simulink model "ofdm_txrx_supermimo"  (Simulink 7.1) (continued)
== system ofdm_txrx_supermimo/EDK Processor == (continued)
      settings: AttributesFormatString=<< %<shared_memory_name> >>  infoedit="Register block that writes data to a shared memory register.  Delay of one sample period."  block_version=10.1.3
  To Register22 : xbsIndex_r4/To Register  shared_memory_name='TxRx_AutoReply_Match0_w'  init=0  ownership=Owned and initialized elsewhere  explicit_data_type=on  arith_type=Unsigned  n_bits=32  bin_pt=0  dbl_ovrd=off  block_type=toreg
      settings: AttributesFormatString=<< %<shared_memory_name> >>  infoedit="Register block that writes data to a shared memory register.  Delay of one sample period."  block_version=10.1.3
  To Register23 : xbsIndex_r4/To Register  shared_memory_name='TxRx_AutoReply_Match1_w'  init=0  ownership=Owned and initialized elsewhere  explicit_data_type=on  arith_type=Unsigned  n_bits=32  bin_pt=0  dbl_ovrd=off  block_type=toreg
      settings: AttributesFormatString=<< %<shared_memory_name> >>  infoedit="Register block that writes data to a shared memory register.  Delay of one sample period."  block_version=10.1.3
  To Register24 : xbsIndex_r4/To Register  shared_memory_name='TxRx_AutoReply_Match2_w'  init=0  ownership=Owned and initialized elsewhere  explicit_data_type=on  arith_type=Unsigned  n_bits=32  bin_pt=0  dbl_ovrd=off  block_type=toreg
      settings: AttributesFormatString=<< %<shared_memory_name> >>  infoedit="Register block that writes data to a shared memory register.  Delay of one sample period."  block_version=10.1.3
  To Register25 : xbsIndex_r4/To Register  shared_memory_name='TxRx_AutoReply_Match3_w'  init=0  ownership=Owned and initialized elsewhere  explicit_data_type=on  arith_type=Unsigned  n_bits=32  bin_pt=0  dbl_ovrd=off  block_type=toreg
      settings: AttributesFormatString=<< %<shared_memory_name> >>  infoedit="Register block that writes data to a shared memory register.  Delay of one sample period."  block_version=10.1.3
  To Register26 : xbsIndex_r4/To Register  shared_memory_name='TxRx_AutoReply_Match4_w'  init=0  ownership=Owned and initialized elsewhere  explicit_data_type=on  arith_type=Unsigned  n_bits=32  bin_pt=0  dbl_ovrd=off  block_type=toreg
      settings: AttributesFormatString=<< %<shared_memory_name> >>  infoedit="Register block that writes data to a shared memory register.  Delay of one sample period."  block_version=10.1.3
  To Register27 : xbsIndex_r4/To Register  shared_memory_name='TxRx_AutoReply_Match5_w'  init=0  ownership=Owned and initialized elsewhere  explicit_data_type=on  arith_type=Unsigned  n_bits=32  bin_pt=0  dbl_ovrd=off  block_type=toreg
      settings: AttributesFormatString=<< %<shared_memory_name> >>  infoedit="Register block that writes data to a shared memory register.  Delay of one sample period."  block_version=10.1.3
  To Register28 : xbsIndex_r4/To Register  shared_memory_name='TxRx_AutoReply_Match6_w'  init=0  ownership=Owned and initialized elsewhere  explicit_data_type=on  arith_type=Unsigned  n_bits=32  bin_pt=0  dbl_ovrd=off  block_type=toreg
      settings: AttributesFormatString=<< %<shared_memory_name> >>  infoedit="Register block that writes data to a shared memory register.  Delay of one sample period."  block_version=10.1.3
  To Register29 : xbsIndex_r4/To Register  shared_memory_name='TxRx_AutoReply_Match7_w'  init=0  ownership=Owned and initialized elsewhere  explicit_data_type=on  arith_type=Unsigned  n_bits=32  bin_pt=0  dbl_ovrd=off  block_type=toreg
      settings: AttributesFormatString=<< %<shared_memory_name> >>  infoedit="Register block that writes data to a shared memory register.  Delay of one sample period."  block_version=10.1.3
  To Register3 : xbsIndex_r4/To Register  shared_memory_name='Rx_Constellation_Scaling_w'  init=0  ownership=Owned and initialized elsewhere  explicit_data_type=on  arith_type=Unsigned  n_bits=32  bin_pt=0  dbl_ovrd=off  block_type=toreg
      settings: AttributesFormatString=<< %<shared_memory_name> >>  infoedit="Register block that writes data to a shared memory register.  Delay of one sample period."  block_version=10.1.3
  To Register30 : xbsIndex_r4/To Register  shared_memory_name='TxRx_FFT_Scaling_w'  init=0  ownership=Owned and initialized elsewhere  explicit_data_type=on  arith_type=Unsigned  n_bits=12  bin_pt=0  dbl_ovrd=off  block_type=toreg
      settings: AttributesFormatString=<< %<shared_memory_name> >>  infoedit="Register block that writes data to a shared memory register.  Delay of one sample period."  block_version=10.1.3
  To Register31 : xbsIndex_r4/To Register  shared_memory_name='TxRx_Interrupt_PktBuf_Ctrl_w'  init=0  ownership=Owned and initialized elsewhere  explicit_data_type=on  arith_type=Unsigned  n_bits=32  bin_pt=0  dbl_ovrd=off  block_type=toreg
      settings: AttributesFormatString=<< %<shared_memory_name> >>  infoedit="Register block that writes data to a shared memory register.  Delay of one sample period."  block_version=10.1.3
  To Register32 : xbsIndex_r4/To Register  shared_memory_name='TxRx_Pilots_Index_w'  init=0  ownership=Owned and initialized elsewhere  explicit_data_type=on  arith_type=Unsigned  n_bits=32  bin_pt=0  dbl_ovrd=off  block_type=toreg
      settings: AttributesFormatString=<< %<shared_memory_name> >>  infoedit="Register block that writes data to a shared memory register.  Delay of one sample period."  block_version=10.1.3
  To Register33 : xbsIndex_r4/To Register  shared_memory_name='TxRx_Pilots_Values_w'  init=0  ownership=Owned and initialized elsewhere  explicit_data_type=on  arith_type=Unsigned  n_bits=32  bin_pt=0  dbl_ovrd=off  block_type=toreg
      settings: AttributesFormatString=<< %<shared_memory_name> >>  infoedit="Register block that writes data to a shared memory register.  Delay of one sample period."  block_version=10.1.3
  To Register34 : xbsIndex_r4/To Register  shared_memory_name='Tx_ControlBits_w'  init=0  ownership=Owned and initialized elsewhere  explicit_data_type=on  arith_type=Unsigned  n_bits=32  bin_pt=0  dbl_ovrd=off  block_type=toreg
      settings: AttributesFormatString=<< %<shared_memory_name> >>  infoedit="Register block that writes data to a shared memory register.  Delay of one sample period."  block_version=10.1.3
  To Register35 : xbsIndex_r4/To Register  shared_memory_name='Tx_Delays_w'  init=0  ownership=Owned and initialized elsewhere  explicit_data_type=on  arith_type=Unsigned  n_bits=32  bin_pt=0  dbl_ovrd=off  block_type=toreg
      settings: AttributesFormatString=<< %<shared_memory_name> >>  infoedit="Register block that writes data to a shared memory register.  Delay of one sample period."  block_version=10.1.3
  To Register36 : xbsIndex_r4/To Register  shared_memory_name='Tx_OFDM_SymCounts_w'  init=0  ownership=Owned and initialized elsewhere  explicit_data_type=on  arith_type=Unsigned  n_bits=32  bin_pt=0  dbl_ovrd=off  block_type=toreg
      settings: AttributesFormatString=<< %<shared_memory_name> >>  infoedit="Register block that writes data to a shared memory register.  Delay of one sample period."  block_version=10.1.3
  To Register37 : xbsIndex_r4/To Register  shared_memory_name='Tx_Scaling_w'  init=0  ownership=Owned and initialized elsewhere  explicit_data_type=on  arith_type=Unsigned  n_bits=32  bin_pt=0  dbl_ovrd=off  block_type=toreg
      settings: AttributesFormatString=<< %<shared_memory_name> >>  infoedit="Register block that writes data to a shared memory register.  Delay of one sample period."  block_version=10.1.3
  To Register38 : xbsIndex_r4/To Register  shared_memory_name='Tx_Start_Reset_Control_w'  init=0  ownership=Owned and initialized elsewhere  explicit_data_type=on  arith_type=Unsigned  n_bits=32  bin_pt=0  dbl_ovrd=off  block_type=toreg
      settings: AttributesFormatString=<< %<shared_memory_name> >>  infoedit="Register block that writes data to a shared memory register.  Delay of one sample period."  block_version=10.1.3
  To Register39 : xbsIndex_r4/To Register  shared_memory_name='pktDet_controlBits_w'  init=0  ownership=Owned and initialized elsewhere  explicit_data_type=on  arith_type=Unsigned  n_bits=32  bin_pt=0  dbl_ovrd=off  block_type=toreg
      settings: AttributesFormatString=<< %<shared_memory_name> >>  infoedit="Register block that writes data to a shared memory register.  Delay of one sample period."  block_version=10.1.3
  To Register4 : xbsIndex_r4/To Register  shared_memory_name='Rx_ControlBits_w'  init=0  ownership=Owned and initialized elsewhere  explicit_data_type=on  arith_type=Unsigned  n_bits=32  bin_pt=0  dbl_ovrd=off  block_type=toreg
      settings: AttributesFormatString=<< %<shared_memory_name> >>  infoedit="Register block that writes data to a shared memory register.  Delay of one sample period."  block_version=10.1.3
  To Register40 : xbsIndex_r4/To Register  shared_memory_name='pktDet_durations_w'  init=0  ownership=Owned and initialized elsewhere  explicit_data_type=on  arith_type=Unsigned  n_bits=32  bin_pt=0  dbl_ovrd=off  block_type=toreg
      settings: AttributesFormatString=<< %<shared_memory_name> >>  infoedit="Register block that writes data to a shared memory register.  Delay of one sample period."  block_version=10.1.3
  To Register41 : xbsIndex_r4/To Register  shared_memory_name='pktDet_thresholds_w'  init=0  ownership=Owned and initialized elsewhere  explicit_data_type=on  arith_type=Unsigned  n_bits=32  bin_pt=0  dbl_ovrd=off  block_type=toreg
      settings: AttributesFormatString=<< %<shared_memory_name> >>  infoedit="Register block that writes data to a shared memory register.  Delay of one sample period."  block_version=10.1.3
  To Register5 : xbsIndex_r4/To Register  shared_memory_name='Rx_FixedPktLen_w'  init=0  ownership=Owned and initialized elsewhere  explicit_data_type=on  arith_type=Unsigned  n_bits=32  bin_pt=0  dbl_ovrd=off  block_type=toreg
      settings: AttributesFormatString=<< %<shared_memory_name> >>  infoedit="Register block that writes data to a shared memory register.  Delay of one sample period."  block_version=10.1.3
  To Register6 : xbsIndex_r4/To Register  shared_memory_name='Rx_OFDM_SymbolCounts_w'  init=0  ownership=Owned and initialized elsewhere  explicit_data_type=on  arith_type=Unsigned  n_bits=32  bin_pt=0  dbl_ovrd=off  block_type=toreg
      settings: AttributesFormatString=<< %<shared_memory_name> >>  infoedit="Register block that writes data to a shared memory register.  Delay of one sample period."  block_version=10.1.3
  To Register7 : xbsIndex_r4/To Register  shared_memory_name='Rx_PilotCalcParams_w'  init=0  ownership=Owned and initialized elsewhere  explicit_data_type=on  arith_type=Unsigned  n_bits=32  bin_pt=0  dbl_ovrd=off  block_type=toreg
      settings: AttributesFormatString=<< %<shared_memory_name> >>  infoedit="Register block that writes data to a shared memory register.  Delay of one sample period."  block_version=10.1.3
  To Register8 : xbsIndex_r4/To Register  shared_memory_name='Rx_PktDet_Delay_w'  init=0  ownership=Owned and initialized elsewhere  explicit_data_type=on  arith_type=Unsigned  n_bits=32  bin_pt=0  dbl_ovrd=off  block_type=toreg
      settings: AttributesFormatString=<< %<shared_memory_name> >>  infoedit="Register block that writes data to a shared memory register.  Delay of one sample period."  block_version=10.1.3
  To Register9 : xbsIndex_r4/To Register  shared_memory_name='Rx_PktDet_LongCorr_Params_w'  init=0  ownership=Owned and initialized elsewhere  explicit_data_type=on  arith_type=Unsigned  n_bits=32  bin_pt=0  dbl_ovrd=off  block_type=toreg
      settings: AttributesFormatString=<< %<shared_memory_name> >>  infoedit="Register block that writes data to a shared memory register.  Delay of one sample period."  block_version=10.1.3
  plb_decode : xbsIndex_r4/MCode  mfname=xlmax  explicit_period=off  period=1  dbl_ovrd=off  enable_stdout=off  enable_debug=off  mfilecontent=|  suppress_output=1  defparams={}  hide_port_list={}  block_type=mcode
      | function [wrDBusReg, addrAck, rdComp, wrDAck, bankAddr, RNWReg, rdDAck, rdDBus, linearAddr] = ...
      |     plb_bus_decode(plbRst, plbABus, plbPAValid, plbRNW, plbWrDBus, rdData, addrPref)
      | 
      | % constant variables (TODO: should pass from outside)
      | ADDRPREF_LEN = 16;
      | BANKADDR_LEN = 2;
      | LINEARADDR_LEN = 12;
      | ABUS_LEN = 32;
      | DBUS_LEN = 32;
      | 
      | % declare and initialize persistent variables
      | % register input bus signals
      | persistent plbRstReg_, plbRstReg_ = xl_state(0, {xlBoolean});
      | persistent plbABusReg_, plbABusReg_ = xl_state(0, {xlUnsigned, ABUS_LEN, 0});
      | persistent plbPAValidReg_, plbPAValidReg_ = xl_state(0, {xlBoolean});
      | persistent plbRNWReg_, plbRNWReg_ = xl_state(0, {xlUnsigned, 1, 0});
      | persistent plbWrDBusReg_, plbWrDBusReg_ = xl_state(0, {xlUnsigned, DBUS_LEN, 0});
      | 
      | % ===== rest of the outputs =====
      | 
      | bankAddr   = xl_slice(plbABusReg_, 2+BANKADDR_LEN+LINEARADDR_LEN-1, 2+LINEARADDR_LEN);
      | linearAddr = xl_slice(plbABusReg_, 2+LINEARADDR_LEN-1, 2);
      | RNWReg = plbRNWReg_;
      | wrDBusReg = plbWrDBusReg_;
      | 
      | % ===== p_select =====
      | 
      | % register PAValid
      | persistent aValidReg, aValidReg = xl_state(0, {xlBoolean});
      | aValidReg = plbPAValidReg_;
      | 
      | % extract and register the address prefix
      | addrPref_in = xl_slice(plbABusReg_, xl_nbits(plbABusReg_)-1, xl_nbits(plbABusReg_)-ADDRPREF_LEN);
      | if addrPref_in == addrPref
      |     ps1 = true;
      | else 
      |     ps1 = false;
      | end 
      | 
      | persistent ps1Reg, ps1Reg = xl_state(0, ps1);
      | ps1Reg = ps1;
      | 
      | ps = xl_and(ps1Reg, aValidReg);
      | 
      | % ===== addrAck =====
      | 
      | % register ps
      | persistent psReg, psReg = xl_state(0, ps);
      | 
      | addrAck = xfix({xlUnsigned, 1, 0}, xl_and(xl_not(plbRstReg_), ps, xl_not(psReg)));
      | 
      | psReg = ps;
      | 
      | % ===== rdComp, rd/wr DAck =====
      |  
      | rdComp1 = xfix({xlUnsigned, 1, 0}, xl_and(addrAck, RNWReg));
      | 
      | NUM_rdCompDelay = 3;
      | persistent rdCompDelay, rdCompDelay = xl_state(zeros(1, NUM_rdCompDelay), rdComp1, NUM_rdCompDelay);
      | rdComp2 = rdCompDelay.back;
      | rdCompDelay.push_front_pop_back(rdComp1);
      | 
      | persistent rdCompReg, rdCompReg = xl_state(0, rdComp1);
      | rdComp = rdCompReg;
      | rdCompReg = rdComp2;
      | 
      | persistent rdDAckReg, rdDAckReg = xl_state(0, rdComp1);
      | rdDAck = rdDAckReg;
      | rdDAckReg = rdComp;
      | 
      | persistent wrDAckReg, wrDAckReg = xl_state(0, addrAck);
      | wrDAck = wrDAckReg;
      | wrDAckReg = xl_and(addrAck, xl_not(RNWReg));
      | 
      | % ===== rdDBus =====
      | 
      | rdSel = xl_or(rdComp2, rdComp);
      | 
      | if rdSel == 1
      |     rdDBus1 = rdData;
      | else
      |     rdDBus1 = 0;
      | end % if
      | 
      | persistent rdDBusReg, rdDBusReg = xl_state(0, rdDBus1);
      | rdDBus = rdDBusReg;
      | rdDBusReg = rdDBus1;
      | 
      | % rdDBus = xl_concat(rdDBus32, rdDBus32);
      | % rdDBus = rdDBus32;
      | 
      | % ===== update the persistent variables =====
      | 
      | plbRstReg_ = plbRst;
      | plbABusReg_ = plbABus;
      | plbPAValidReg_ = plbPAValid;
      | plbRNWReg_ = plbRNW;
      | plbWrDBusReg_ = xl_slice(plbWrDBus, DBUS_LEN-1, 0);
      settings: block_version=10.1.3
  plb_memmap : xbsIndex_r4/MCode  mfname=xlmax  explicit_period=off  period=1  dbl_ovrd=off  enable_stdout=off  enable_debug=off  mfilecontent=|  suppress_output=1  defparams={}  hide_port_list={}  block_type=mcode
      | function [read_bank_out, sm_Rx_AF_Blanking_w_din, sm_Rx_AF_Blanking_w_en, sm_Rx_AF_TxScaling_w_din, sm_Rx_AF_TxScaling_w_en, sm_Rx_ChanEst_MinMag_w_din, sm_Rx_ChanEst_MinMag_w_en, sm_Rx_Constellation_Scaling_w_din, sm_Rx_Constellation_Scaling_w_en, sm_Rx_ControlBits_w_din, sm_Rx_ControlBits_w_en, sm_Rx_FixedPktLen_w_din, sm_Rx_FixedPktLen_w_en, sm_Rx_OFDM_SymbolCounts_w_din, sm_Rx_OFDM_SymbolCounts_w_en, sm_Rx_PilotCalcParams_w_din, sm_Rx_PilotCalcParams_w_en, sm_Rx_PktDet_Delay_w_din, sm_Rx_PktDet_Delay_w_en, sm_Rx_PktDet_LongCorr_Params_w_din, sm_Rx_PktDet_LongCorr_Params_w_en, sm_Rx_PktDet_LongCorr_Thresholds_w_din, sm_Rx_PktDet_LongCorr_Thresholds_w_en, sm_Rx_PreCFO_Options_w_din, sm_Rx_PreCFO_Options_w_en, sm_Rx_PreCFO_PilotCalcCorrection_w_din, sm_Rx_PreCFO_PilotCalcCorrection_w_en, sm_Rx_coarseCFO_correction_w_din, sm_Rx_coarseCFO_correction_w_en, sm_Rx_pktByteNums_w_din, sm_Rx_pktByteNums_w_en, sm_Rx_pktDet_Tresholds_w_din, sm_Rx_pktDet_Tresholds_w_en, sm_TxRx_AutoReply_Action0_w_din, sm_TxRx_AutoReply_Action0_w_en, sm_TxRx_AutoReply_Action1_w_din, sm_TxRx_AutoReply_Action1_w_en, sm_TxRx_AutoReply_Action2_w_din, sm_TxRx_AutoReply_Action2_w_en, sm_TxRx_AutoReply_Action3_w_din, sm_TxRx_AutoReply_Action3_w_en, sm_TxRx_AutoReply_Action4_w_din, sm_TxRx_AutoReply_Action4_w_en, sm_TxRx_AutoReply_Action5_w_din, sm_TxRx_AutoReply_Action5_w_en, sm_TxRx_AutoReply_Match0_w_din, sm_TxRx_AutoReply_Match0_w_en, sm_TxRx_AutoReply_Match1_w_din, sm_TxRx_AutoReply_Match1_w_en, sm_TxRx_AutoReply_Match2_w_din, sm_TxRx_AutoReply_Match2_w_en, sm_TxRx_AutoReply_Match3_w_din, sm_TxRx_AutoReply_Match3_w_en, sm_TxRx_AutoReply_Match4_w_din, sm_TxRx_AutoReply_Match4_w_en, sm_TxRx_AutoReply_Match5_w_din, sm_TxRx_AutoReply_Match5_w_en, sm_TxRx_AutoReply_Match6_w_din, sm_TxRx_AutoReply_Match6_w_en, sm_TxRx_AutoReply_Match7_w_din, sm_TxRx_AutoReply_Match7_w_en, sm_TxRx_FFT_Scaling_w_din, sm_TxRx_FFT_Scaling_w_en, sm_TxRx_Interrupt_PktBuf_Ctrl_w_din, sm_TxRx_Interrupt_PktBuf_Ctrl_w_en, sm_TxRx_Pilots_Index_w_din, sm_TxRx_Pilots_Index_w_en, sm_TxRx_Pilots_Values_w_din, sm_TxRx_Pilots_Values_w_en, sm_Tx_ControlBits_w_din, sm_Tx_ControlBits_w_en, sm_Tx_Delays_w_din, sm_Tx_Delays_w_en, sm_Tx_OFDM_SymCounts_w_din, sm_Tx_OFDM_SymCounts_w_en, sm_Tx_Scaling_w_din, sm_Tx_Scaling_w_en, sm_Tx_Start_Reset_Control_w_din, sm_Tx_Start_Reset_Control_w_en, sm_pktDet_controlBits_w_din, sm_pktDet_controlBits_w_en, sm_pktDet_durations_w_din, sm_pktDet_durations_w_en, sm_pktDet_thresholds_w_din, sm_pktDet_thresholds_w_en, sm_ChannelEstimates_addr, sm_ChannelEstimates_din, sm_ChannelEstimates_we, sm_EVM_perSC_addr, sm_EVM_perSC_din, sm_EVM_perSC_we, sm_EVM_perSym_addr, sm_EVM_perSym_din, sm_EVM_perSym_we, sm_PktBufFreqOffsets_addr, sm_PktBufFreqOffsets_din, sm_PktBufFreqOffsets_we, sm_RxModulation_addr, sm_RxModulation_din, sm_RxModulation_we, sm_TxHeaderTranslate_addr, sm_TxHeaderTranslate_din, sm_TxHeaderTranslate_we, sm_TxModulation_addr, sm_TxModulation_din, sm_TxModulation_we] = plb_memmap(wrDBus, bankAddr, linearAddr, RNWReg, addrAck, sm_Rx_BER_Errors, sm_Rx_BER_TotalBits, sm_Rx_ControlBits_r, sm_Rx_Gains, sm_Rx_OFDM_SymbolCounts_r, sm_Rx_PktDet_Delay_r, sm_Rx_PktDet_LongCorr_Params_r, sm_Rx_coarseCFOest, sm_Rx_pilotCFOest, sm_Rx_pktByteNums_r, sm_Rx_pktDetEventCount, sm_Rx_pktDone_interruptStatus, sm_TxRx_AutoReply_Action0_r, sm_TxRx_AutoReply_Action1_r, sm_TxRx_AutoReply_Action2_r, sm_TxRx_AutoReply_Action3_r, sm_TxRx_AutoReply_Action4_r, sm_TxRx_AutoReply_Action5_r, sm_TxRx_AutoReply_Match0_r, sm_TxRx_AutoReply_Match1_r, sm_TxRx_AutoReply_Match2_r, sm_TxRx_AutoReply_Match3_r, sm_TxRx_AutoReply_Match4_r, sm_TxRx_AutoReply_Match5_r, sm_TxRx_AutoReply_Match6_r, sm_TxRx_AutoReply_Match7_r, sm_TxRx_Interrupt_PktBuf_Ctrl_r, sm_Tx_ControlBits_r, sm_Tx_Delays_r, sm_Tx_OFDM_SymCounts_r, sm_Tx_PktRunning, sm_Tx_Start_Reset_Control_r, sm_midPacketRSSI, sm_pktDet_controlBits_r, sm_pktDet_durations_r, sm_pktDet_status, sm_pktDet_thresholds_r, sm_ChannelEstimates, sm_EVM_perSC, sm_EVM_perSym, sm_PktBufFreqOffsets, sm_RxModulation, sm_TxHeaderTranslate, sm_TxModulation)
      | 
      | 
      | % connvert the input data to UFix_32_0 (the bus data type)
      | % 'From Register' blocks
      | % sm_Rx_BER_Errors_bus = xfix({xlUnsigned, 32, 0}, 0);
      | sm_Rx_BER_Errors_bus = xl_force(sm_Rx_BER_Errors, xlUnsigned, 0);
      | 
      | % sm_Rx_BER_TotalBits_bus = xfix({xlUnsigned, 32, 0}, 0);
      | sm_Rx_BER_TotalBits_bus = xl_force(sm_Rx_BER_TotalBits, xlUnsigned, 0);
      | 
      | % sm_Rx_ControlBits_r_bus = xfix({xlUnsigned, 32, 0}, 0);
      | sm_Rx_ControlBits_r_bus = xl_force(sm_Rx_ControlBits_r, xlUnsigned, 0);
      | 
      | % sm_Rx_Gains_bus = xfix({xlUnsigned, 32, 0}, 0);
      | sm_Rx_Gains_bus = xl_force(sm_Rx_Gains, xlUnsigned, 0);
      | 
      | % sm_Rx_OFDM_SymbolCounts_r_bus = xfix({xlUnsigned, 32, 0}, 0);
      | sm_Rx_OFDM_SymbolCounts_r_bus = xl_force(sm_Rx_OFDM_SymbolCounts_r, xlUnsigned, 0);
      | 
      | % sm_Rx_PktDet_Delay_r_bus = xfix({xlUnsigned, 32, 0}, 0);
      | sm_Rx_PktDet_Delay_r_bus = xl_force(sm_Rx_PktDet_Delay_r, xlUnsigned, 0);
      | 
      | % sm_Rx_PktDet_LongCorr_Params_r_bus = xfix({xlUnsigned, 32, 0}, 0);
      | sm_Rx_PktDet_LongCorr_Params_r_bus = xl_force(sm_Rx_PktDet_LongCorr_Params_r, xlUnsigned, 0);
      | 
      | % sm_Rx_coarseCFOest_bus = xfix({xlUnsigned, 32, 0}, 0);
      | sm_Rx_coarseCFOest_bus = xl_force(sm_Rx_coarseCFOest, xlUnsigned, 0);
      | 
      | % sm_Rx_pilotCFOest_bus = xfix({xlUnsigned, 32, 0}, 0);
      | sm_Rx_pilotCFOest_bus = xl_force(sm_Rx_pilotCFOest, xlUnsigned, 0);
      | 
      | % sm_Rx_pktByteNums_r_bus = xfix({xlUnsigned, 32, 0}, 0);
      | sm_Rx_pktByteNums_r_bus = xl_force(sm_Rx_pktByteNums_r, xlUnsigned, 0);
      | 
      | % sm_Rx_pktDetEventCount_bus = xfix({xlUnsigned, 32, 0}, 0);
      | sm_Rx_pktDetEventCount_bus = xl_force(sm_Rx_pktDetEventCount, xlUnsigned, 0);
      | 
      | % sm_Rx_pktDone_interruptStatus_bus = xfix({xlUnsigned, 32, 0}, 0);
      | sm_Rx_pktDone_interruptStatus_bus = xl_force(sm_Rx_pktDone_interruptStatus, xlUnsigned, 0);
      | 
      | % sm_TxRx_AutoReply_Action0_r_bus = xfix({xlUnsigned, 32, 0}, 0);
      | sm_TxRx_AutoReply_Action0_r_bus = xl_force(sm_TxRx_AutoReply_Action0_r, xlUnsigned, 0);
      | 
      | % sm_TxRx_AutoReply_Action1_r_bus = xfix({xlUnsigned, 32, 0}, 0);
      | sm_TxRx_AutoReply_Action1_r_bus = xl_force(sm_TxRx_AutoReply_Action1_r, xlUnsigned, 0);
      | 
      | % sm_TxRx_AutoReply_Action2_r_bus = xfix({xlUnsigned, 32, 0}, 0);
      | sm_TxRx_AutoReply_Action2_r_bus = xl_force(sm_TxRx_AutoReply_Action2_r, xlUnsigned, 0);
      | 
      | % sm_TxRx_AutoReply_Action3_r_bus = xfix({xlUnsigned, 32, 0}, 0);
      | sm_TxRx_AutoReply_Action3_r_bus = xl_force(sm_TxRx_AutoReply_Action3_r, xlUnsigned, 0);
      | 
      | % sm_TxRx_AutoReply_Action4_r_bus = xfix({xlUnsigned, 32, 0}, 0);
      | sm_TxRx_AutoReply_Action4_r_bus = xl_force(sm_TxRx_AutoReply_Action4_r, xlUnsigned, 0);
      | 
      | % sm_TxRx_AutoReply_Action5_r_bus = xfix({xlUnsigned, 32, 0}, 0);
      | sm_TxRx_AutoReply_Action5_r_bus = xl_force(sm_TxRx_AutoReply_Action5_r, xlUnsigned, 0);
      | 
      | % sm_TxRx_AutoReply_Match0_r_bus = xfix({xlUnsigned, 32, 0}, 0);
      | sm_TxRx_AutoReply_Match0_r_bus = xl_force(sm_TxRx_AutoReply_Match0_r, xlUnsigned, 0);
      | 
      | % sm_TxRx_AutoReply_Match1_r_bus = xfix({xlUnsigned, 32, 0}, 0);
      | sm_TxRx_AutoReply_Match1_r_bus = xl_force(sm_TxRx_AutoReply_Match1_r, xlUnsigned, 0);
      | 
      | % sm_TxRx_AutoReply_Match2_r_bus = xfix({xlUnsigned, 32, 0}, 0);
      | sm_TxRx_AutoReply_Match2_r_bus = xl_force(sm_TxRx_AutoReply_Match2_r, xlUnsigned, 0);
      | 
      | % sm_TxRx_AutoReply_Match3_r_bus = xfix({xlUnsigned, 32, 0}, 0);
      | sm_TxRx_AutoReply_Match3_r_bus = xl_force(sm_TxRx_AutoReply_Match3_r, xlUnsigned, 0);
      | 
      | % sm_TxRx_AutoReply_Match4_r_bus = xfix({xlUnsigned, 32, 0}, 0);
      | sm_TxRx_AutoReply_Match4_r_bus = xl_force(sm_TxRx_AutoReply_Match4_r, xlUnsigned, 0);
      | 
      | % sm_TxRx_AutoReply_Match5_r_bus = xfix({xlUnsigned, 32, 0}, 0);
      | sm_TxRx_AutoReply_Match5_r_bus = xl_force(sm_TxRx_AutoReply_Match5_r, xlUnsigned, 0);
      | 
      | % sm_TxRx_AutoReply_Match6_r_bus = xfix({xlUnsigned, 32, 0}, 0);
      | sm_TxRx_AutoReply_Match6_r_bus = xl_force(sm_TxRx_AutoReply_Match6_r, xlUnsigned, 0);
      | 
      | % sm_TxRx_AutoReply_Match7_r_bus = xfix({xlUnsigned, 32, 0}, 0);
      | sm_TxRx_AutoReply_Match7_r_bus = xl_force(sm_TxRx_AutoReply_Match7_r, xlUnsigned, 0);
      | 
      | % sm_TxRx_Interrupt_PktBuf_Ctrl_r_bus = xfix({xlUnsigned, 32, 0}, 0);
      | sm_TxRx_Interrupt_PktBuf_Ctrl_r_bus = xl_force(sm_TxRx_Interrupt_PktBuf_Ctrl_r, xlUnsigned, 0);
      | 
      | % sm_Tx_ControlBits_r_bus = xfix({xlUnsigned, 32, 0}, 0);
      | sm_Tx_ControlBits_r_bus = xl_force(sm_Tx_ControlBits_r, xlUnsigned, 0);
      | 
      | % sm_Tx_Delays_r_bus = xfix({xlUnsigned, 32, 0}, 0);
      | sm_Tx_Delays_r_bus = xl_force(sm_Tx_Delays_r, xlUnsigned, 0);
      | 
      | % sm_Tx_OFDM_SymCounts_r_bus = xfix({xlUnsigned, 32, 0}, 0);
      | sm_Tx_OFDM_SymCounts_r_bus = xl_force(sm_Tx_OFDM_SymCounts_r, xlUnsigned, 0);
      | 
      | % sm_Tx_PktRunning_bus = xfix({xlUnsigned, 32, 0}, 0);
      | sm_Tx_PktRunning_bus = xl_force(sm_Tx_PktRunning, xlUnsigned, 0);
      | 
      | % sm_Tx_Start_Reset_Control_r_bus = xfix({xlUnsigned, 32, 0}, 0);
      | sm_Tx_Start_Reset_Control_r_bus = xl_force(sm_Tx_Start_Reset_Control_r, xlUnsigned, 0);
      | 
      | % sm_midPacketRSSI_bus = xfix({xlUnsigned, 32, 0}, 0);
      | sm_midPacketRSSI_bus = xl_force(sm_midPacketRSSI, xlUnsigned, 0);
      | 
      | % sm_pktDet_controlBits_r_bus = xfix({xlUnsigned, 32, 0}, 0);
      | sm_pktDet_controlBits_r_bus = xl_force(sm_pktDet_controlBits_r, xlUnsigned, 0);
      | 
      | % sm_pktDet_durations_r_bus = xfix({xlUnsigned, 32, 0}, 0);
      | sm_pktDet_durations_r_bus = xl_force(sm_pktDet_durations_r, xlUnsigned, 0);
      | 
      | % sm_pktDet_status_bus = xfix({xlUnsigned, 32, 0}, 0);
      | sm_pktDet_status_bus = xl_force(sm_pktDet_status, xlUnsigned, 0);
      | 
      | % sm_pktDet_thresholds_r_bus = xfix({xlUnsigned, 32, 0}, 0);
      | sm_pktDet_thresholds_r_bus = xl_force(sm_pktDet_thresholds_r, xlUnsigned, 0);
      | 
      | % 'To Register' blocks
      | 
      | % 'From FIFO' blocks
      | % 'To FIFO' blocks
      | % 'Shared Memory' blocks
      | % ChannelEstimates_bus = xfix({xlUnsigned, 32, 0}, 0);
      | sm_ChannelEstimates_bus = xl_force(sm_ChannelEstimates, xlUnsigned, 0);
      | 
      | % EVM_perSC_bus = xfix({xlUnsigned, 32, 0}, 0);
      | sm_EVM_perSC_bus = xl_force(sm_EVM_perSC, xlUnsigned, 0);
      | 
      | % EVM_perSym_bus = xfix({xlUnsigned, 32, 0}, 0);
      | sm_EVM_perSym_bus = xl_force(sm_EVM_perSym, xlUnsigned, 0);
      | 
      | % PktBufFreqOffsets_bus = xfix({xlUnsigned, 32, 0}, 0);
      | sm_PktBufFreqOffsets_bus = xl_force(sm_PktBufFreqOffsets, xlUnsigned, 0);
      | 
      | % RxModulation_bus = xfix({xlUnsigned, 32, 0}, 0);
      | sm_RxModulation_bus = xl_force(sm_RxModulation, xlUnsigned, 0);
      | 
      | % TxHeaderTranslate_bus = xfix({xlUnsigned, 32, 0}, 0);
      | sm_TxHeaderTranslate_bus = xl_force(sm_TxHeaderTranslate, xlUnsigned, 0);
      | 
      | % TxModulation_bus = xfix({xlUnsigned, 32, 0}, 0);
      | sm_TxModulation_bus = xl_force(sm_TxModulation, xlUnsigned, 0);
      | 
      | 
      | % 'dout' ports of 'From Register' blocks
      | 
      | % registered register mux output
      | persistent reg_bank_out_reg; reg_bank_out_reg = xl_state(0, {xlUnsigned, 32, 0});
      | reg_bank_out = reg_bank_out_reg;
      | 
      | if linearAddr == 0
      |     reg_bank_out_reg = sm_Rx_BER_Errors_bus;
      | elseif linearAddr == 1
      |     reg_bank_out_reg = sm_Rx_BER_TotalBits_bus;
      | elseif linearAddr == 2
      |     reg_bank_out_reg = sm_Rx_ControlBits_r_bus;
      | elseif linearAddr == 3
      |     reg_bank_out_reg = sm_Rx_Gains_bus;
      | elseif linearAddr == 4
      |     reg_bank_out_reg = sm_Rx_OFDM_SymbolCounts_r_bus;
      | elseif linearAddr == 5
      |     reg_bank_out_reg = sm_Rx_PktDet_Delay_r_bus;
      | elseif linearAddr == 6
      |     reg_bank_out_reg = sm_Rx_PktDet_LongCorr_Params_r_bus;
      | elseif linearAddr == 7
      |     reg_bank_out_reg = sm_Rx_coarseCFOest_bus;
      | elseif linearAddr == 8
      |     reg_bank_out_reg = sm_Rx_pilotCFOest_bus;
      | elseif linearAddr == 9
      |     reg_bank_out_reg = sm_Rx_pktByteNums_r_bus;
      | elseif linearAddr == 10
      |     reg_bank_out_reg = sm_Rx_pktDetEventCount_bus;
      | elseif linearAddr == 11
      |     reg_bank_out_reg = sm_Rx_pktDone_interruptStatus_bus;
      | elseif linearAddr == 12
      |     reg_bank_out_reg = sm_TxRx_AutoReply_Action0_r_bus;
      | elseif linearAddr == 13
      |     reg_bank_out_reg = sm_TxRx_AutoReply_Action1_r_bus;
      | elseif linearAddr == 14
      |     reg_bank_out_reg = sm_TxRx_AutoReply_Action2_r_bus;
      | elseif linearAddr == 15
      |     reg_bank_out_reg = sm_TxRx_AutoReply_Action3_r_bus;
      | elseif linearAddr == 16
      |     reg_bank_out_reg = sm_TxRx_AutoReply_Action4_r_bus;
      | elseif linearAddr == 17
      |     reg_bank_out_reg = sm_TxRx_AutoReply_Action5_r_bus;
      | elseif linearAddr == 18
      |     reg_bank_out_reg = sm_TxRx_AutoReply_Match0_r_bus;
      | elseif linearAddr == 19
      |     reg_bank_out_reg = sm_TxRx_AutoReply_Match1_r_bus;
      | elseif linearAddr == 20
      |     reg_bank_out_reg = sm_TxRx_AutoReply_Match2_r_bus;
      | elseif linearAddr == 21
      |     reg_bank_out_reg = sm_TxRx_AutoReply_Match3_r_bus;
      | elseif linearAddr == 22
      |     reg_bank_out_reg = sm_TxRx_AutoReply_Match4_r_bus;
      | elseif linearAddr == 23
      |     reg_bank_out_reg = sm_TxRx_AutoReply_Match5_r_bus;
      | elseif linearAddr == 24
      |     reg_bank_out_reg = sm_TxRx_AutoReply_Match6_r_bus;
      | elseif linearAddr == 25
      |     reg_bank_out_reg = sm_TxRx_AutoReply_Match7_r_bus;
      | elseif linearAddr == 26
      |     reg_bank_out_reg = sm_TxRx_Interrupt_PktBuf_Ctrl_r_bus;
      | elseif linearAddr == 27
      |     reg_bank_out_reg = sm_Tx_ControlBits_r_bus;
      | elseif linearAddr == 28
      |     reg_bank_out_reg = sm_Tx_Delays_r_bus;
      | elseif linearAddr == 29
      |     reg_bank_out_reg = sm_Tx_OFDM_SymCounts_r_bus;
      | elseif linearAddr == 30
      |     reg_bank_out_reg = sm_Tx_PktRunning_bus;
      | elseif linearAddr == 31
      |     reg_bank_out_reg = sm_Tx_Start_Reset_Control_r_bus;
      | elseif linearAddr == 32
      |     reg_bank_out_reg = sm_midPacketRSSI_bus;
      | elseif linearAddr == 33
      |     reg_bank_out_reg = sm_pktDet_controlBits_r_bus;
      | elseif linearAddr == 34
      |     reg_bank_out_reg = sm_pktDet_durations_r_bus;
      | elseif linearAddr == 35
      |     reg_bank_out_reg = sm_pktDet_status_bus;
      | elseif linearAddr == 36
      |     reg_bank_out_reg = sm_pktDet_thresholds_r_bus;
      | 
      | end
      | 
      | 
      | % 'From FIFO' and 'To FIFO' blocks
      | 
      | 
      | 
      | 
      | 
      | opCode = xl_concat(addrAck, RNWReg, bankAddr, linearAddr);
      | 
      | % 'Shared Memory' blocks
      | 
      | sm_ChannelEstimates_sel_value = xl_concat(xl_slice(linearAddr, ...
      |                                         xl_nbits(linearAddr) - 1, ...
      |                                         8) ...
      |                                     );
      | if sm_ChannelEstimates_sel_value == xfix({xlUnsigned, ...
      |                                         xl_nbits(linearAddr) - 8, ...
      |                                         0}, ...
      |                                         4);
      |     sm_ChannelEstimates_sel = true;
      | else
      |     sm_ChannelEstimates_sel = false;
      | end
      | sm_EVM_perSC_sel_value = xl_concat(xl_slice(linearAddr, ...
      |                                         xl_nbits(linearAddr) - 1, ...
      |                                         6) ...
      |                                     );
      | if sm_EVM_perSC_sel_value == xfix({xlUnsigned, ...
      |                                         xl_nbits(linearAddr) - 6, ...
      |                                         0}, ...
      |                                         32);
      |     sm_EVM_perSC_sel = true;
      | else
      |     sm_EVM_perSC_sel = false;
      | end
      | sm_EVM_perSym_sel_value = xl_concat(xl_slice(linearAddr, ...
      |                                         xl_nbits(linearAddr) - 1, ...
      |                                         8) ...
      |                                     );
      | if sm_EVM_perSym_sel_value == xfix({xlUnsigned, ...
      |                                         xl_nbits(linearAddr) - 8, ...
      |                                         0}, ...
      |                                         5);
      |     sm_EVM_perSym_sel = true;
      | else
      |     sm_EVM_perSym_sel = false;
      | end
      | sm_PktBufFreqOffsets_sel_value = xl_concat(xl_slice(linearAddr, ...
      |                                         xl_nbits(linearAddr) - 1, ...
      |                                         5) ...
      |                                     );
      | if sm_PktBufFreqOffsets_sel_value == xfix({xlUnsigned, ...
      |                                         xl_nbits(linearAddr) - 5, ...
      |                                         0}, ...
      |                                         66);
      |     sm_PktBufFreqOffsets_sel = true;
      | else
      |     sm_PktBufFreqOffsets_sel = false;
      | end
      | sm_RxModulation_sel_value = xl_concat(xl_slice(linearAddr, ...
      |                                         xl_nbits(linearAddr) - 1, ...
      |                                         8) ...
      |                                     );
      | if sm_RxModulation_sel_value == xfix({xlUnsigned, ...
      |                                         xl_nbits(linearAddr) - 8, ...
      |                                         0}, ...
      |                                         6);
      |     sm_RxModulation_sel = true;
      | else
      |     sm_RxModulation_sel = false;
      | end
      | sm_TxHeaderTranslate_sel_value = xl_concat(xl_slice(linearAddr, ...
      |                                         xl_nbits(linearAddr) - 1, ...
      |                                         10) ...
      |                                     );
      | if sm_TxHeaderTranslate_sel_value == xfix({xlUnsigned, ...
      |                                         xl_nbits(linearAddr) - 10, ...
      |                                         0}, ...
      |                                         0);
      |     sm_TxHeaderTranslate_sel = true;
      | else
      |     sm_TxHeaderTranslate_sel = false;
      | end
      | sm_TxModulation_sel_value = xl_concat(xl_slice(linearAddr, ...
      |                                         xl_nbits(linearAddr) - 1, ...
      |                                         8) ...
      |                                     );
      | if sm_TxModulation_sel_value == xfix({xlUnsigned, ...
      |                                         xl_nbits(linearAddr) - 8, ...
      |                                         0}, ...
      |                                         7);
      |     sm_TxModulation_sel = true;
      | else
      |     sm_TxModulation_sel = false;
      | end
      | 
      | 
      | % registered Shared Memory mux output
      | persistent ram_bank_out_reg; ram_bank_out_reg = xl_state(0, {xlUnsigned, 32, 0});
      | ram_bank_out = ram_bank_out_reg;
      | if sm_ChannelEstimates_sel
      |     ram_bank_out_reg = sm_ChannelEstimates_bus;
      | elseif sm_EVM_perSC_sel
      |     ram_bank_out_reg = sm_EVM_perSC_bus;
      | elseif sm_EVM_perSym_sel
      |     ram_bank_out_reg = sm_EVM_perSym_bus;
      | elseif sm_PktBufFreqOffsets_sel
      |     ram_bank_out_reg = sm_PktBufFreqOffsets_bus;
      | elseif sm_RxModulation_sel
      |     ram_bank_out_reg = sm_RxModulation_bus;
      | elseif sm_TxHeaderTranslate_sel
      |     ram_bank_out_reg = sm_TxHeaderTranslate_bus;
      | elseif sm_TxModulation_sel
      |     ram_bank_out_reg = sm_TxModulation_bus;
      | end
      | 
      | % 'din' ports of 'Shared Memory' blocks
      | sm_ChannelEstimates_din = xl_force(xl_slice(wrDBus, 32 - 1, 0), ...
      |                                  xlUnsigned, ...
      |                                  0);
      | sm_EVM_perSC_din = xl_force(xl_slice(wrDBus, 32 - 1, 0), ...
      |                                  xlSigned, ...
      |                                  22);
      | sm_EVM_perSym_din = xl_force(xl_slice(wrDBus, 32 - 1, 0), ...
      |                                  xlSigned, ...
      |                                  14);
      | sm_PktBufFreqOffsets_din = xl_force(xl_slice(wrDBus, 32 - 1, 0), ...
      |                                  xlUnsigned, ...
      |                                  32);
      | sm_RxModulation_din = xl_force(xl_slice(wrDBus, 4 - 1, 0), ...
      |                                  xlUnsigned, ...
      |                                  0);
      | sm_TxHeaderTranslate_din = xl_force(xl_slice(wrDBus, 10 - 1, 0), ...
      |                                  xlUnsigned, ...
      |                                  0);
      | sm_TxModulation_din = xl_force(xl_slice(wrDBus, 4 - 1, 0), ...
      |                                  xlUnsigned, ...
      |                                  0);
      | 
      | 
      | % 'we' ports of 'Shared Memory' blocks
      | persistent sm_ChannelEstimates_we_reg; sm_ChannelEstimates_we_reg = xl_state(false, {xlBoolean});
      | sm_ChannelEstimates_we = sm_ChannelEstimates_we_reg;
      | opCode_sm_ChannelEstimates = xl_concat(addrAck, ...
      |                                      RNWReg, ...
      |                                      bankAddr, ...
      |                                      xl_slice(linearAddr, ...
      |                                               xl_nbits(linearAddr) - 1, ...
      |                                               8) ...
      |                                     );
      | if opCode_sm_ChannelEstimates == xl_concat(xfix({xlUnsigned, 4, 0}, 8), ...
      |                                          xfix({xlUnsigned, ...
      |                                                xl_nbits(linearAddr) - 8, ...
      |                                                0}, ...
      |                                                4) ...
      |                                         );
      |     sm_ChannelEstimates_we_reg = true;
      | else
      |     sm_ChannelEstimates_we_reg = false;
      | end
      | persistent sm_EVM_perSC_we_reg; sm_EVM_perSC_we_reg = xl_state(false, {xlBoolean});
      | sm_EVM_perSC_we = sm_EVM_perSC_we_reg;
      | opCode_sm_EVM_perSC = xl_concat(addrAck, ...
      |                                      RNWReg, ...
      |                                      bankAddr, ...
      |                                      xl_slice(linearAddr, ...
      |                                               xl_nbits(linearAddr) - 1, ...
      |                                               6) ...
      |                                     );
      | if opCode_sm_EVM_perSC == xl_concat(xfix({xlUnsigned, 4, 0}, 8), ...
      |                                          xfix({xlUnsigned, ...
      |                                                xl_nbits(linearAddr) - 6, ...
      |                                                0}, ...
      |                                                32) ...
      |                                         );
      |     sm_EVM_perSC_we_reg = true;
      | else
      |     sm_EVM_perSC_we_reg = false;
      | end
      | persistent sm_EVM_perSym_we_reg; sm_EVM_perSym_we_reg = xl_state(false, {xlBoolean});
      | sm_EVM_perSym_we = sm_EVM_perSym_we_reg;
      | opCode_sm_EVM_perSym = xl_concat(addrAck, ...
      |                                      RNWReg, ...
      |                                      bankAddr, ...
      |                                      xl_slice(linearAddr, ...
      |                                               xl_nbits(linearAddr) - 1, ...
      |                                               8) ...
      |                                     );
      | if opCode_sm_EVM_perSym == xl_concat(xfix({xlUnsigned, 4, 0}, 8), ...
      |                                          xfix({xlUnsigned, ...
      |                                                xl_nbits(linearAddr) - 8, ...
      |                                                0}, ...
      |                                                5) ...
      |                                         );
      |     sm_EVM_perSym_we_reg = true;
      | else
      |     sm_EVM_perSym_we_reg = false;
      | end
      | persistent sm_PktBufFreqOffsets_we_reg; sm_PktBufFreqOffsets_we_reg = xl_state(false, {xlBoolean});
      | sm_PktBufFreqOffsets_we = sm_PktBufFreqOffsets_we_reg;